simulink model "TosAeb"  (R2021a)
settings: Solver=FixedStepDiscrete  StartTime=0.0  StopTime=inf  FixedStep=0.01  RelTol=1e-3
undefined symbols (not defined in the file or in its folder): ACCModSts, AccSts1, AEB_ObjectMovingOrientation, AEB_ObjectMovingState, CN_BTCActive, CN_CurrentGearR, CN_CurrentGearR_DHT, CN_PTCActive, CN_VDCActive, CN_VSC_EspOff, CN_VSC_FCW_AEB_Error, CN_VSC_FCW_AEB_NoDispay, CN_VSC_FCW_AEB_PerfmDgdtn, CN_VSC_FCW_AEB_TempUnAvlbl, CN_VSC_VehStandstill, CNaccelAvoidMax, CNaccelAvoidMin, CNbrakePedalPress, CNcomfortStrLeftAccelMax, CNcomfortStrLeftAccelMin, CNcomfortStrRightAccelMax, CNcomfortStrRightAccelMin, CNDrvDoorOpen, CNDrvModeEco, CNDrvModeNorm, CNDrvModeOther, CNDrvModeSport, CNDrvSBRreminder, CNNull, CNobjInvalidID, CNPassDoorOpen, CNPassSBRreminder, CNPEBSAccelNegInf, CNPEBSAccelNull, CNPEBSTimeInf, CNPEBSTimeNull, CNSTOPMAX, CNSTOPNULL, CNsubjWidth, CNSwtOFF, CNSwtON, CNTTCMAX, CNTTCNULL, CNVehConfigAEBCycNone, CNVehConfigAEBNone, CNVehConfigAEBPedNone, CNVehConfigFCWNone, CNVehConfigJANone, CollisionSeverity, FALSE, FCWSnvty, JACycObjCalcInfoDefaultParams, JAPedObjCalcInfoDefaultParams, JAVehObjCalcInfoDefaultParams, latCycObjCalcInfoDefaultParams, latPedObjCalcInfoDefaultParams, longObjCalcInfoDefaultParams, ModSts, movingStatus, ObjectType ... (+1112)
libraries: simulink x3031, AEBSFunctionLib x50, Ta_Library x33, AEBSStateMachineLib x23, Mylibrary x12, simulink_extras x7, ObjSelect_lib x3, dspstat3 x2
(part 63 of 102)

== system TosAeb/FcwAebJa_Step_sys/ALG_AEBSystem/ALG_AEBController/AEB_LatObjInfoCalcApp/LatPedObjCalc/LatPedObjCalc/latPedObjInfoCalc/ObjColiisionRiskProbCalc/CollisionRiskProbCalc/EgoReginCorrCalc ==
  dxReginEgoRU : Inport  OutDataTypeStr=single
  dyReginEgoRU : Inport  Port=2
  dxReginEgoLL : Inport  Port=3  OutDataTypeStr=single
  dyReginEgoLL : Inport  Port=4
  Add : Sum  OutDataTypeStr=single
  Add1 : Sum  Inputs=+-  OutDataTypeStr=single
  Add2 : Sum  OutDataTypeStr=single
  ObjectLength : Constant  Value=0.5  OutDataTypeStr=single
  ObjectWidth : Constant  Value=0.5  OutDataTypeStr=single
  dxReginEgoRUCorr : Outport
  dyReginEgoRUCorr : Outport  Port=2
  dyReginEgoLLCorr : Outport  Port=3
  dxReginEgoLLCorr : Outport  Port=4
  wires:
  Add/1 -> dxReginEgoRUCorr/1
  dyReginEgoRU/1 -> Add1/1
  ObjectWidth/1 -> Add2/1, Add1/2
  Add1/1 -> dyReginEgoRUCorr/1
  dxReginEgoLL/1 -> dxReginEgoLLCorr/1
  dyReginEgoLL/1 -> Add2/2
  Add2/1 -> dyReginEgoLLCorr/1
  dxReginEgoRU/1 -> Add/2
  ObjectLength/1 -> Add/1

== system TosAeb/FcwAebJa_Step_sys/ALG_AEBSystem/ALG_AEBController/AEB_LatObjInfoCalcApp/LatPedObjCalc/LatPedObjCalc/latPedObjInfoCalc/ObjColiisionRiskProbCalc/CollisionRiskProbCalc/ObjAbsInfoCalc ==
  vx : Inport
  vy : Inport  Port=2
  dy : Inport  Port=3
  dxFrontBumper : Inport  Port=4
  subjYawRate : Inport  Port=5
  subjVel : Inport  Port=6
  Bus Creator1 : BusCreator  Inputs=2
  RadicalRelevantCalc : Subsystem
  RadicalToXYTrans : Subsystem
  XYToRadicalTrans : Subsystem
  sincosCoefCalc : Subsystem
  velAbsObjCorr : Outport
  wires:
  RadicalRelevantCalc/1(radialVelRel) -> XYToRadicalTrans/2(radialVelRel)  "radialVelRel"
  subjYawRate/1 -> RadicalRelevantCalc/3(subjYawRate)
  dy/1 -> RadicalRelevantCalc/1(dyTargetObjCalc)
  sincosCoefCalc/1(sincosCoef) -> XYToRadicalTrans/3(sincosCoef), RadicalToXYTrans/3(sincosCoef)
  vx/1 -> Bus Creator1/1  "vxRelTargetObjCalc"
  subjVel/1 -> RadicalToXYTrans/1(subjVel)
  dxFrontBumper/1 -> RadicalRelevantCalc/2(dxTargetObjCalc)
  Bus Creator1/1 -> XYToRadicalTrans/1(LongLatVelRel)  "LongLatVelRel"
  vy/1 -> Bus Creator1/2  "vyRelTargetObjCalc"
  XYToRadicalTrans/1(velRadicalXY) -> RadicalToXYTrans/2(velRadicalXY)
  RadicalRelevantCalc/2(objAngleToRear) -> sincosCoefCalc/1(angle)  "objAngleToRear"
  RadicalToXYTrans/1(velAbsObjCorr) -> velAbsObjCorr/1

== system TosAeb/FcwAebJa_Step_sys/ALG_AEBSystem/ALG_AEBController/AEB_LatObjInfoCalcApp/LatPedObjCalc/LatPedObjCalc/latPedObjInfoCalc/ObjColiisionRiskProbCalc/CollisionRiskProbCalc/ObjReginCorrCalc ==
  velAbsObjCorr : Inport
  tPredTime : Inport  Port=2  OutDataTypeStr=single
  ObjectTypeCameraConfirmed : Inport  Port=3
  ObjectLength : Inport  Port=4
  ObjectWidth : Inport  Port=5
  ObjectYawAngle : Inport  Port=6
  ObjectMovingOrientation : Inport  Port=7
  dx : Inport  Port=8
  dy : Inport  Port=9
  AccelXYMaxMinCorrCalc : Subsystem
  ObjFrameCalc : Subsystem
  ObjLengthWidthCalc : Subsystem
  ObjReginCorrCalc : Subsystem
  PedMaxAccelCalc : Subsystem
  dxReginObjRUCorr : Outport
  dyReginObjRUCorr : Outport  Port=2
  dxReginObjLLCorr : Outport  Port=3
  dyReginObjLLCorr : Outport  Port=4
  wires:
  AccelXYMaxMinCorrCalc/1(AccelXMaxMinCorr) -> ObjReginCorrCalc/1(AccelXMaxMinCorr)
  velAbsObjCorr/1 -> PedMaxAccelCalc/1(velAbsObjCorr), AccelXYMaxMinCorrCalc/2(velAbsTargetCorr), ObjReginCorrCalc/3(velAbsTargetCorr)
  PedMaxAccelCalc/1(maxAccelDecel) -> AccelXYMaxMinCorrCalc/1(maxAccelDecel)
  ObjFrameCalc/1(dxMax) -> ObjReginCorrCalc/4(dxMax)
  ObjFrameCalc/3(dyMax) -> ObjReginCorrCalc/6(dyMax)
  AccelXYMaxMinCorrCalc/2(AccelYMaxMinCorr) -> ObjReginCorrCalc/2(AccelYMaxMinCorr)
  tPredTime/1 -> PedMaxAccelCalc/2(tPredTime), [tPredTime]
  ObjFrameCalc/2(dxMin) -> ObjReginCorrCalc/5(dxMin)
  ObjFrameCalc/4(dyMin) -> ObjReginCorrCalc/7(dyMin)
  [tPredTime] -> ObjReginCorrCalc/8(tPredTime)
  dx/1 -> ObjFrameCalc/1(dx)
  dy/1 -> ObjFrameCalc/2(dy)
  ObjLengthWidthCalc/1(ObjLengthCorr) -> ObjFrameCalc/3(ObjLengthCorr)
  ObjLengthWidthCalc/2(ObjWidthCorr) -> ObjFrameCalc/4(ObjWidthCorr)
  ObjReginCorrCalc/1(dxReginObjRUCorr) -> dxReginObjRUCorr/1
  ObjReginCorrCalc/2(dyReginObjRUCorr) -> dyReginObjRUCorr/1
  ObjReginCorrCalc/3(dxReginObjLLCorr) -> dxReginObjLLCorr/1
  ObjReginCorrCalc/4(dyReginObjLLCorr) -> dyReginObjLLCorr/1
  ObjectMovingOrientation/1 -> ObjLengthWidthCalc/5(ObjectMovingOrientation)
  ObjectYawAngle/1 -> ObjLengthWidthCalc/4(ObjectYawAngle)
  ObjectWidth/1 -> ObjLengthWidthCalc/3(ObjectWidth)
  ObjectLength/1 -> ObjLengthWidthCalc/2(ObjectLength)
  ObjectTypeCameraConfirmed/1 -> ObjLengthWidthCalc/1(ObjectTypeCameraConfirmed)

== system TosAeb/FcwAebJa_Step_sys/ALG_AEBSystem/ALG_AEBController/AEB_LatObjInfoCalcApp/LatPedObjCalc/LatPedObjCalc/latPedObjInfoCalc/ObjCrossFlgCal/PedPreCollisionCalcFunc/ObjBoundingBox ==
  ObjLengthCorr : Inport  OutDataTypeStr=single
  ObjWidthCorr : Inport  Port=2  OutDataTypeStr=single
  dxPreRefPointObj : Inport  Port=3  OutDataTypeStr=single
  dyPreRefPointObj : Inport  Port=4  OutDataTypeStr=single
  sincosCoef : Inport  Port=5
  Bus Creator : BusCreator  Inputs=5
  Mux : Mux
  Mux1 : Mux
  ObjLLCoordinate : Subsystem
  ObjLUCoordinate : Subsystem
  ObjRLCoordinate : Subsystem
  ObjRUCoordinate : Subsystem
  objDxPre : Outport
  objDyPre : Outport  Port=2
  wires:
  ObjLengthCorr/1 -> Bus Creator/1  "ObjLengthCorr"
  ObjWidthCorr/1 -> Bus Creator/2  "ObjWidthCorr"
  dxPreRefPointObj/1 -> Bus Creator/3  "dxPreRefPointObj"
  dyPreRefPointObj/1 -> Bus Creator/4  "dyPreRefPointObj"
  sincosCoef/1 -> Bus Creator/5  "sincosCoef"
  Bus Creator/1 -> ObjRLCoordinate/1(inputSignals), ObjRUCoordinate/1(inputSignals), ObjLLCoordinate/1(inputSignals), ObjLUCoordinate/1(inputSignals)
  ObjLUCoordinate/1(dxPreLUObj) -> [dxPreLUObj]  "dxPreLUObj"
  ObjLUCoordinate/2(dyPreLUObj) -> [dyPreLUObj]  "dyPreLUObj"
  ObjLLCoordinate/1(dxPre_LLObj) -> [dxPreLLObj]  "dxPreLLObj"
  ObjLLCoordinate/2(dyPreLLObj) -> [dyPreLLObj]  "dyPreLLObj"
  ObjRUCoordinate/1(dxPreRUObj) -> [dxPreRUObj]  "dxPreRUObj"
  ObjRUCoordinate/2(dyPreRUObj) -> [dyPreRUObj]  "dyPreRUObj"
  ObjRLCoordinate/1(dxPreRLObj) -> [dxPreRLObj]  "dxPreRLObj"
  ObjRLCoordinate/2(dyPreRLObj) -> [dyPreRLObj]  "dyPreRLObj"
  Mux/1 -> objDxPre/1
  Mux1/1 -> objDyPre/1
  [dxPreRUObj] -> Mux/1  "dxPreRUObj"
  [dxPreLUObj] -> Mux/2  "dxPreLUObj"
  [dxPreLLObj] -> Mux/3  "dxPreLLObj"
  [dxPreRLObj] -> Mux/4  "dxPreRLObj"
  [dyPreRUObj] -> Mux1/1  "dyPreRUObj"
  [dyPreLUObj] -> Mux1/2  "dyPreLUObj"
  [dyPreLLObj] -> Mux1/3  "dyPreLLObj"
  [dyPreRLObj] -> Mux1/4  "dyPreRLObj"

== system TosAeb/FcwAebJa_Step_sys/ALG_AEBSystem/ALG_AEBController/AEB_LatObjInfoCalcApp/LatPedObjCalc/LatPedObjCalc/latPedObjInfoCalc/ObjCrossFlgCal/PedPreCollisionCalcFunc/ObjLenthWidthCalc ==
  ObjectYawAngle : Inport
  ObjectMovingOrientation : Inport  Port=2
  ObjectTypeCameraConfirmed : Inport  Port=3
  ObjectLength : Inport  Port=4
  ObjectWidth : Inport  Port=5
  ObjLengthWidthCalc : Stateflow chart  TreatAsAtomicUnit=on
  P_LatPedCA_PreCollisionPedLengthThres : Constant  Value=P_AEB_LatPedCA_PreCollisionPedLengthThres
  P_LatPedCA_PreCollisionPedWidthThres : Constant  Value=P_AEB_LatPedCA_PreCollisionPedWidthThres
  ObjLengthCorr : Outport
  ObjWidthCorr : Outport  Port=2
  wires:
  ObjectYawAngle/1 -> ObjLengthWidthCalc/1(ObjectYawAngle)
  ObjectLength/1 -> ObjLengthWidthCalc/4(ObjectLengthIn)
  ObjectWidth/1 -> ObjLengthWidthCalc/5(ObjectWidthIn)
  ObjLengthWidthCalc/1(ObjectLength) -> ObjLengthCorr/1
  ObjLengthWidthCalc/2(ObjectWidth) -> ObjWidthCorr/1
  ObjectMovingOrientation/1 -> ObjLengthWidthCalc/2(ObjectMovingOrientation)
  P_LatPedCA_PreCollisionPedLengthThres/1 -> ObjLengthWidthCalc/6(P_LatPedCA_PreCollisionPedLengthThres)
  P_LatPedCA_PreCollisionPedWidthThres/1 -> ObjLengthWidthCalc/7(P_LatPedCA_PreCollisionPedWidthThres)
  ObjectTypeCameraConfirmed/1 -> ObjLengthWidthCalc/3(ObjectTypeCameraConfirmed)

== system TosAeb/FcwAebJa_Step_sys/ALG_AEBSystem/ALG_AEBController/AEB_LatObjInfoCalcApp/LatPedObjCalc/LatPedObjCalc/latPedObjInfoCalc/ObjCrossFlgCal/PedPreCollisionCalcFunc/ObjPreReferPointCalc ==
  dx : Inport
  vxAbsObj : Inport  Port=2
  tPredTime : Inport  Port=3  OutDataTypeStr=single
  axAbsObj : Inport  Port=4
  vy : Inport  Port=5
  subjLatAccel : Inport  Port=6
  ay : Inport  Port=7
  dy : Inport  Port=8
  ObjectYawAngle : Inport  Port=9
  Add : Sum  Inputs=+++  OutDataTypeStr=single
  Add1 : Sum  Inputs=+++  OutDataTypeStr=single
  Add2 : Sum
  Degrees to Radians : simulink_extras/Transformations/Degrees to Radians
  Gain : Gain  Gain=0.5  OutDataTypeStr=single
  Gain1 : Gain  Gain=0.5  OutDataTypeStr=single
  Math Function : Math  Operator=square  SignedPower=on  OutDataTypeStr=single  SaturateOnIntegerOverflow=on
  Math Function1 : Math  Operator=square  SignedPower=on  OutDataTypeStr=single  SaturateOnIntegerOverflow=on
  Product{,1..3} (4 alike) : Product  OutDataTypeStr=single
  dxPreRefPointObj : Outport
  dyPreRefPointObj : Outport  Port=2
  YawAnglePreObj : Outport  Port=3
  wires:
  vxAbsObj/1 -> Product/1
  tPredTime/1 -> Product/2, Math Function1/1, Product2/2, Math Function/1
  Product/1 -> Add/2
  Math Function/1 -> Product1/1
  axAbsObj/1 -> Product1/2
  Product1/1 -> Gain/1
  Gain/1 -> Add/3
  Add/1 -> dxPreRefPointObj/1
  dx/1 -> Add/1
  vy/1 -> Product2/1
  Math Function1/1 -> Product3/1
  Product3/1 -> Gain1/1
  Product2/1 -> Add1/1
  Gain1/1 -> Add1/2
  dy/1 -> Add1/3
  Add1/1 -> dyPreRefPointObj/1
  ObjectYawAngle/1 -> Degrees to Radians/1
  subjLatAccel/1 -> Add2/1
  ay/1 -> Add2/2
  Add2/1 -> Product3/2
  Degrees to Radians/1 -> YawAnglePreObj/1

== system TosAeb/FcwAebJa_Step_sys/ALG_AEBSystem/ALG_AEBController/AEB_LatObjInfoCalcApp/LatPedObjCalc/LatPedObjCalc/latPedObjInfoCalc/ObjCrossFlgCal/PedPreCollisionCalcFunc/PreCrossFlgCalcFlow/VectorCrossAlgorithm ==
  x1 : Inport  OutDataTypeStr=single
  y1 : Inport  Port=2  OutDataTypeStr=single
  x2 : Inport  Port=3  OutDataTypeStr=single
  y2 : Inport  Port=4  OutDataTypeStr=single
  m1 : Inport  Port=5  OutDataTypeStr=single
  n1 : Inport  Port=6  OutDataTypeStr=single
  m2 : Inport  Port=7  OutDataTypeStr=single
  n2 : Inport  Port=8  OutDataTypeStr=single
  f : TriggerPort  TriggerType=function-call  VariantControl=Variant
      settings: CopyFcn=Stateflow.SLINSF.SimulinkMan.removeBlockPreDeleteFcn  PreDeleteFcn=Stateflow.SLINSF.SimulinkMan.unsafePredeleteFcn
  Abs : Abs
  Bus Creator : BusCreator  Inputs=8
  Bus Selector : BusSelector  OutputSignals=x2,x1,n2,n1,y2,y1,m2,m1
  Compare To Constant : simulink/Logic and Bit Operations/Compare To Constant  relop=<  const=1e-6  OutDataTypeStr=boolean
  If : If  IfExpression=u1
  If Action Subsystem : Subsystem  TreatAsAtomicUnit=on
  If Action Subsystem1 : Subsystem  TreatAsAtomicUnit=on
  Merge : Merge
  VectorMulit : Subsystem
  pedPreCollisionFlg : Outport
  wires:
  x1/1 -> Bus Creator/1  "x1"
  y1/1 -> Bus Creator/2  "y1"
  x2/1 -> Bus Creator/3  "x2"
  y2/1 -> Bus Creator/4  "y2"
  m1/1 -> Bus Creator/5  "m1"
  n1/1 -> Bus Creator/6  "n1"
  m2/1 -> Bus Creator/7  "m2"
  n2/1 -> Bus Creator/8  "n2"
  Bus Creator/1 -> If Action Subsystem1/2(Coordinations), Bus Selector/1
  VectorMulit/1(vectorMulit) -> If Action Subsystem1/1(vectorMulit), Abs/1
  Abs/1 -> Compare To Constant/1
  Compare To Constant/1 -> If/1
  If/1 -> If Action Subsystem/Action
  If/2 -> If Action Subsystem1/Action
  If Action Subsystem/1(CrossFlg) -> Merge/1
  If Action Subsystem1/1(CrossFlg) -> Merge/2
  Merge/1 -> pedPreCollisionFlg/1
  Bus Selector/2 -> VectorMulit/2(x1)  "<x1>"
  Bus Selector/1 -> VectorMulit/1(x2)  "<x2>"
  Bus Selector/3 -> VectorMulit/3(n2)  "<n2>"
  Bus Selector/4 -> VectorMulit/4(n1)  "<n1>"
  Bus Selector/5 -> VectorMulit/5(y2)  "<y2>"
  Bus Selector/6 -> VectorMulit/6(y1)  "<y1>"
  Bus Selector/7 -> VectorMulit/7(m2)  "<m2>"
  Bus Selector/8 -> VectorMulit/8(m1)  "<m1>"

== system TosAeb/FcwAebJa_Step_sys/ALG_AEBSystem/ALG_AEBController/AEB_LatObjInfoCalcApp/LatPedObjCalc/LatPedObjCalc/latPedObjInfoCalc/ObjCrossFlgCal/PedPreCollisionCalcFunc/PreRefPointEgoCalc ==
  subjVel : Inport
  tPredTime : Inport  Port=2  OutDataTypeStr=single
  subjLongAccel : Inport  Port=3
  subjLatAccel : Inport  Port=4
  subjYawRate : Inport  Port=5
  Add : Sum  OutDataTypeStr=single
  Gain : Gain  Gain=0.5  OutDataTypeStr=single
  Gain1 : Gain  Gain=0.5  OutDataTypeStr=single
  Math Function : Math  Operator=square  SignedPower=on  OutDataTypeStr=single  SaturateOnIntegerOverflow=on
  Product{,1..3} (4 alike) : Product  OutDataTypeStr=single
  " dxPreRefPoint" : Outport
  " dyPreRefPoint" : Outport  Port=2
  YawAnglePre : Outport  Port=3
  wires:
  Gain1/1 -> " dyPreRefPoint"/1
  Gain/1 -> Add/2
  Product3/1 -> YawAnglePre/1
  subjYawRate/1 -> Product3/2
  Product2/1 -> Gain1/1
  subjLatAccel/1 -> Product2/2
  Add/1 -> " dxPreRefPoint"/1
  subjLongAccel/1 -> Product1/2
  Math Function/1 -> Product1/1, Product2/1
  tPredTime/1 -> Math Function/1, Product3/1, Product/2
  subjVel/1 -> Product/1
  Product/1 -> Add/1
  Product1/1 -> Gain/1

== system TosAeb/FcwAebJa_Step_sys/ALG_AEBSystem/ALG_AEBController/AEB_LatObjInfoCalcApp/LatPedObjCalc/LatPedObjCalc/latPedObjInfoCalc/ObjCrossFlgCal/PedPreCollisionCalcFunc/SubjBoundingBox ==
  dxPreRefPoint : Inport  OutDataTypeStr=single
  dyPreRefPoint : Inport  Port=2  OutDataTypeStr=single
  sincosCoef : Inport  Port=3
  Abs : Abs
  Bus Creator : BusCreator  Inputs=6
  Constant : Constant  Value=P_Global_DxvFrontBumper_m
  Constant1 : Constant  Value=P_Global_DxvRearBumper_m
  Constant2 : Constant  Value=P_Global_VehicleWidthMin_m
  Mux : Mux
  Mux1 : Mux
  SubjLLCoordinate : Subsystem
  SubjLUCoordinate : Subsystem
  SubjRLCoordinate : Subsystem
  SubjRUCoordinate : Subsystem
  subjDxPre : Outport
  subjDyPre : Outport  Port=2
  wires:
  Constant2/1 -> Bus Creator/6  "subjWidth"
  Abs/1 -> Bus Creator/5  "dxRearBumperToRearAxis"
  Constant/1 -> Bus Creator/4  "dxFrontBumperToRearAxis"
  sincosCoef/1 -> Bus Creator/3  "sincosCoef"
  dyPreRefPoint/1 -> Bus Creator/2  "dyPreRefPoint"
  dxPreRefPoint/1 -> Bus Creator/1  "dxPreRefPoint"
  Bus Creator/1 -> SubjRLCoordinate/1(inputSignals), SubjRUCoordinate/1(inputSignals), SubjLLCoordinate/1(inputSignals), SubjLUCoordinate/1(inputSignals)
  SubjLUCoordinate/1(dxPreLU) -> [dxPreLU]  "dxPreLU"
  SubjLUCoordinate/2(dyPreLU) -> [dyPreLU]  "dyPreLU"
  SubjLLCoordinate/1(dxPreLL) -> [dxPreLL]  "dxPreLL"
  SubjLLCoordinate/2(dyPreLL) -> [dyPreLL]  "dyPreLL"
  SubjRUCoordinate/1(dxPreRU) -> [dxPreRU]  "dxPreRU"
  SubjRUCoordinate/2(dyPreRU) -> [dyPreRU]  "dyPreRU"
  SubjRLCoordinate/1(dxPreRL) -> [dxPreRL]  "dxPreRL"
  SubjRLCoordinate/2(dyPreRL) -> [dyPreRL]  "dyPreRL"
  Mux/1 -> subjDxPre/1
  Mux1/1 -> subjDyPre/1
  [dxPreRU] -> Mux/1
  [dxPreLU] -> Mux/2
  [dxPreLL] -> Mux/3
  [dxPreRL] -> Mux/4
  [dyPreRU] -> Mux1/1
  [dyPreLU] -> Mux1/2
  [dyPreLL] -> Mux1/3
  [dyPreRL] -> Mux1/4
  Constant1/1 -> Abs/1

== system TosAeb/FcwAebJa_Step_sys/ALG_AEBSystem/ALG_AEBController/AEB_LatObjInfoCalcApp/LatPedObjCalc/LatPedObjCalc/latPedObjInfoCalc/ObjCrossFlgCal/PedPreCollisionCalcFunc/sincosCoefCalc ==
  angle : Inport  OutDataTypeStr=single
  Bus Creator : BusCreator  Inputs=2
  Sincos : Trigonometry  Operator=sincos
  sincosCoef : Outport
  wires:
  Sincos/1 -> Bus Creator/1  "sinCoef"
  angle/1 -> Sincos/1
  Sincos/2 -> Bus Creator/2  "cosCoef"
  Bus Creator/1 -> sincosCoef/1  "sincosCoef"

== system TosAeb/FcwAebJa_Step_sys/ALG_AEBSystem/ALG_AEBController/AEB_LatObjInfoCalcApp/LatPedObjCalc/LatPedObjCalc/latPedObjInfoCalc/ObjCrossFlgCal/PedPreCollisionCalcFunc/sincosCoefCalc1 ==
  angle : Inport
  Bus Creator : BusCreator  Inputs=2
  Sincos : Trigonometry  Operator=sincos
  sincosCoef : Outport
  wires:
  Bus Creator/1 -> sincosCoef/1  "sincosCoef"
  Sincos/2 -> Bus Creator/2  "cosCoef"
  angle/1 -> Sincos/1
  Sincos/1 -> Bus Creator/1  "sinCoef"

== system TosAeb/FcwAebJa_Step_sys/ALG_AEBSystem/ALG_AEBController/AEB_LatObjInfoCalcApp/LatPedObjCalc/Subsystem/latPedObjInfoCalc/ObjColiisionRiskProbCalc/CollisionRiskProbCalc/CollisionRiskProbCalc ==
  dxReginEgoRUCorr : Inport  OutDataTypeStr=single
  dyReginEgoRUCorr : Inport  Port=2  OutDataTypeStr=single
  dyReginEgoLLCorr : Inport  Port=3  OutDataTypeStr=single
  dxReginEgoLLCorr : Inport  Port=4  OutDataTypeStr=single
  dxReginObjRUCorr : Inport  Port=5  OutDataTypeStr=single
  dyReginObjRUCorr : Inport  Port=6  OutDataTypeStr=single
  dxReginObjLLCorr : Inport  Port=7  OutDataTypeStr=single
  dyReginObjLLCorr : Inport  Port=8  OutDataTypeStr=single
  Bus Creator : BusCreator  Inputs=8
  Bus Selector : BusSelector  OutputSignals=dyReginEgoRUCorr,dyReginEgoLLCorr,dyReginObjRUCorr,dyReginObjLLCorr
  Bus Selector1 : BusSelector  OutputSignals=dxReginEgoRUCorr,dxReginEgoLLCorr,dxReginObjRUCorr,dxReginObjLLCorr
  Bus Selector2 : BusSelector  OutputSignals=dxReginObjLLCorr,dyReginObjRUCorr,dyReginObjLLCorr
  CriticalityPointCalc : Subsystem
  dxOverlapFlow : Stateflow chart  TreatAsAtomicUnit=on
  dyOverlapFlow : Stateflow chart  TreatAsAtomicUnit=on
  pedCollisionRiskProbCalc : Subsystem
  dxPreCollison : Outport
  dyPreCollison : Outport  Port=2
  collisionRiskProb : Outport  Port=3
  dyCollisionRiskProb : Outport  Port=4
  dxCollisionRiskProb : Outport  Port=5
  wires:
  dyOverlapFlow/1(dyOverlap) -> pedCollisionRiskProbCalc/1(dyOverlap)
  dyOverlapFlow/2(dyOverlapLimit) -> pedCollisionRiskProbCalc/2(dyOverlapLimit)
  dxOverlapFlow/1(dxOverlap) -> pedCollisionRiskProbCalc/3(dxOverlap)
  dxOverlapFlow/2(dxOverlapLimit) -> pedCollisionRiskProbCalc/4(dxOverlapLimit)
  pedCollisionRiskProbCalc/1(collisionRiskProb) -> collisionRiskProb/1
  dyReginEgoLLCorr/1 -> Bus Creator/3  "dyReginEgoLLCorr"
  dyReginEgoRUCorr/1 -> Bus Creator/2  "dyReginEgoRUCorr"
  dxReginEgoLLCorr/1 -> Bus Creator/4  "dxReginEgoLLCorr"
  dxReginObjRUCorr/1 -> Bus Creator/5  "dxReginObjRUCorr"
  dxReginEgoRUCorr/1 -> Bus Creator/1  "dxReginEgoRUCorr"
  dyReginObjRUCorr/1 -> Bus Creator/6  "dyReginObjRUCorr"
  dxReginObjLLCorr/1 -> Bus Creator/7  "dxReginObjLLCorr"
  dyReginObjLLCorr/1 -> Bus Creator/8  "dyReginObjLLCorr"
  Bus Creator/1 -> Bus Selector1/1, Bus Selector/1, Bus Selector2/1
  Bus Selector/1 -> dyOverlapFlow/1(dyReginEgoRUCorr)  "<dyReginEgoRUCorr>"
  Bus Selector/2 -> dyOverlapFlow/2(dyReginEgoLLCorr)  "<dyReginEgoLLCorr>"
  Bus Selector1/1 -> dxOverlapFlow/1(dxReginEgoRUCorr)  "<dxReginEgoRUCorr>"
  Bus Selector1/2 -> dxOverlapFlow/2(dxReginEgoLLCorr)  "<dxReginEgoLLCorr>"
  Bus Selector2/1 -> CriticalityPointCalc/1(dxReginObjLLCorr)  "<dxReginObjLLCorr>"
  Bus Selector2/2 -> CriticalityPointCalc/2(dyReginObjRUCorr)  "<dyReginObjRUCorr>"
  Bus Selector2/3 -> CriticalityPointCalc/3(dyReginObjLLCorr)  "<dyReginObjLLCorr>"
  CriticalityPointCalc/1(dxPreCollison) -> dxPreCollison/1
  CriticalityPointCalc/2(dyPreCollison) -> dyPreCollison/1
  Bus Selector/3 -> dyOverlapFlow/3(dyReginObjRUCorr)  "<dyReginObjRUCorr>"
  Bus Selector/4 -> dyOverlapFlow/4(dyReginObjLLCorr)  "<dyReginObjLLCorr>"
  Bus Selector1/3 -> dxOverlapFlow/3(dxReginObjRUCorr)  "<dxReginObjRUCorr>"
  Bus Selector1/4 -> dxOverlapFlow/4(dxReginObjLLCorr)  "<dxReginObjLLCorr>"
  pedCollisionRiskProbCalc/2(dyCollisionRiskProb) -> dyCollisionRiskProb/1
  pedCollisionRiskProbCalc/3(dxCollisionRiskProb) -> dxCollisionRiskProb/1

== system TosAeb/FcwAebJa_Step_sys/ALG_AEBSystem/ALG_AEBController/AEB_LatObjInfoCalcApp/LatPedObjCalc/Subsystem/latPedObjInfoCalc/ObjColiisionRiskProbCalc/CollisionRiskProbCalc/EgoReginCalc ==
  tPredTime : Inport  OutDataTypeStr=single
  subjVel : Inport  Port=2
  Abs : Abs
  Add : Sum  OutDataTypeStr=single
  Add1 : Sum  Inputs=+-  OutDataTypeStr=single
  Constant : Constant  Value=P_Global_DxvRearBumper_m
  Constant1 : Constant  Value=P_Global_VehicleWidthMin_m
  Constant2 : Constant  Value=P_Global_DxvFrontBumper_m
  Gain : Gain  Gain=-0.5
  Gain1 : Gain  Gain=0.5
  Product : Product  OutDataTypeStr=single
  dxReginEgoRU : Outport
  dyReginEgoRU : Outport  Port=2
  dxReginEgoLL : Outport  Port=3
  dyReginEgoLL : Outport  Port=4
  wires:
  Product/1 -> Add/1, Add1/1
  Constant2/1 -> Add/2
  Add/1 -> dxReginEgoRU/1
  Constant1/1 -> Gain1/1, Gain/1
  Gain/1 -> dyReginEgoRU/1
  Gain1/1 -> dyReginEgoLL/1
  Constant/1 -> Abs/1
  Add1/1 -> dxReginEgoLL/1
  tPredTime/1 -> Product/1
  subjVel/1 -> Product/2
  Abs/1 -> Add1/2

== system TosAeb/FcwAebJa_Step_sys/ALG_AEBSystem/ALG_AEBController/AEB_LatObjInfoCalcApp/LatPedObjCalc/Subsystem/latPedObjInfoCalc/ObjColiisionRiskProbCalc/CollisionRiskProbCalc/EgoReginCorrCalc ==
  dxReginEgoRU : Inport  OutDataTypeStr=single
  dyReginEgoRU : Inport  Port=2
  dxReginEgoLL : Inport  Port=3  OutDataTypeStr=single
  dyReginEgoLL : Inport  Port=4
  Add : Sum  OutDataTypeStr=single
  Add1 : Sum  Inputs=+-  OutDataTypeStr=single
  Add2 : Sum  OutDataTypeStr=single
  ObjectLength : Constant  Value=0.5  OutDataTypeStr=single
  ObjectWidth : Constant  Value=0.5  OutDataTypeStr=single
  dxReginEgoRUCorr : Outport
  dyReginEgoRUCorr : Outport  Port=2
  dyReginEgoLLCorr : Outport  Port=3
  dxReginEgoLLCorr : Outport  Port=4
  wires:
  Add/1 -> dxReginEgoRUCorr/1
  dyReginEgoRU/1 -> Add1/1
  ObjectWidth/1 -> Add2/1, Add1/2
  Add1/1 -> dyReginEgoRUCorr/1
  dxReginEgoLL/1 -> dxReginEgoLLCorr/1
  dyReginEgoLL/1 -> Add2/2
  Add2/1 -> dyReginEgoLLCorr/1
  dxReginEgoRU/1 -> Add/2
  ObjectLength/1 -> Add/1

== system TosAeb/FcwAebJa_Step_sys/ALG_AEBSystem/ALG_AEBController/AEB_LatObjInfoCalcApp/LatPedObjCalc/Subsystem/latPedObjInfoCalc/ObjColiisionRiskProbCalc/CollisionRiskProbCalc/ObjAbsInfoCalc ==
  vx : Inport
  vy : Inport  Port=2
  dy : Inport  Port=3
  dxFrontBumper : Inport  Port=4
  subjYawRate : Inport  Port=5
  subjVel : Inport  Port=6
  Bus Creator1 : BusCreator  Inputs=2
  RadicalRelevantCalc : Subsystem
  RadicalToXYTrans : Subsystem
  XYToRadicalTrans : Subsystem
  sincosCoefCalc : Subsystem
  velAbsObjCorr : Outport
  wires:
  RadicalRelevantCalc/1(radialVelRel) -> XYToRadicalTrans/2(radialVelRel)  "radialVelRel"
  subjYawRate/1 -> RadicalRelevantCalc/3(subjYawRate)
  dy/1 -> RadicalRelevantCalc/1(dyTargetObjCalc)
  sincosCoefCalc/1(sincosCoef) -> XYToRadicalTrans/3(sincosCoef), RadicalToXYTrans/3(sincosCoef)
  vx/1 -> Bus Creator1/1  "vxRelTargetObjCalc"
  subjVel/1 -> RadicalToXYTrans/1(subjVel)
  dxFrontBumper/1 -> RadicalRelevantCalc/2(dxTargetObjCalc)
  Bus Creator1/1 -> XYToRadicalTrans/1(LongLatVelRel)  "LongLatVelRel"
  vy/1 -> Bus Creator1/2  "vyRelTargetObjCalc"
  XYToRadicalTrans/1(velRadicalXY) -> RadicalToXYTrans/2(velRadicalXY)
  RadicalRelevantCalc/2(objAngleToRear) -> sincosCoefCalc/1(angle)  "objAngleToRear"
  RadicalToXYTrans/1(velAbsObjCorr) -> velAbsObjCorr/1

== system TosAeb/FcwAebJa_Step_sys/ALG_AEBSystem/ALG_AEBController/AEB_LatObjInfoCalcApp/LatPedObjCalc/Subsystem/latPedObjInfoCalc/ObjColiisionRiskProbCalc/CollisionRiskProbCalc/ObjReginCorrCalc ==
  velAbsObjCorr : Inport
  tPredTime : Inport  Port=2  OutDataTypeStr=single
  ObjectTypeCameraConfirmed : Inport  Port=3
  ObjectLength : Inport  Port=4
  ObjectWidth : Inport  Port=5
  ObjectYawAngle : Inport  Port=6
  ObjectMovingOrientation : Inport  Port=7
  dx : Inport  Port=8
  dy : Inport  Port=9
  AccelXYMaxMinCorrCalc : Subsystem
  ObjFrameCalc : Subsystem
  ObjLengthWidthCalc : Subsystem
  ObjReginCorrCalc : Subsystem
  PedMaxAccelCalc : Subsystem
  dxReginObjRUCorr : Outport
  dyReginObjRUCorr : Outport  Port=2
  dxReginObjLLCorr : Outport  Port=3
  dyReginObjLLCorr : Outport  Port=4
  wires:
  AccelXYMaxMinCorrCalc/1(AccelXMaxMinCorr) -> ObjReginCorrCalc/1(AccelXMaxMinCorr)
  velAbsObjCorr/1 -> PedMaxAccelCalc/1(velAbsObjCorr), AccelXYMaxMinCorrCalc/2(velAbsTargetCorr), ObjReginCorrCalc/3(velAbsTargetCorr)
  PedMaxAccelCalc/1(maxAccelDecel) -> AccelXYMaxMinCorrCalc/1(maxAccelDecel)
  ObjFrameCalc/1(dxMax) -> ObjReginCorrCalc/4(dxMax)
  ObjFrameCalc/3(dyMax) -> ObjReginCorrCalc/6(dyMax)
  AccelXYMaxMinCorrCalc/2(AccelYMaxMinCorr) -> ObjReginCorrCalc/2(AccelYMaxMinCorr)
  tPredTime/1 -> PedMaxAccelCalc/2(tPredTime), [tPredTime]
  ObjFrameCalc/2(dxMin) -> ObjReginCorrCalc/5(dxMin)
  ObjFrameCalc/4(dyMin) -> ObjReginCorrCalc/7(dyMin)
  [tPredTime] -> ObjReginCorrCalc/8(tPredTime)
  dx/1 -> ObjFrameCalc/1(dx)
  dy/1 -> ObjFrameCalc/2(dy)
  ObjLengthWidthCalc/1(ObjLengthCorr) -> ObjFrameCalc/3(ObjLengthCorr)
  ObjLengthWidthCalc/2(ObjWidthCorr) -> ObjFrameCalc/4(ObjWidthCorr)
  ObjReginCorrCalc/1(dxReginObjRUCorr) -> dxReginObjRUCorr/1
  ObjReginCorrCalc/2(dyReginObjRUCorr) -> dyReginObjRUCorr/1
  ObjReginCorrCalc/3(dxReginObjLLCorr) -> dxReginObjLLCorr/1
  ObjReginCorrCalc/4(dyReginObjLLCorr) -> dyReginObjLLCorr/1
  ObjectMovingOrientation/1 -> ObjLengthWidthCalc/5(ObjectMovingOrientation)
  ObjectYawAngle/1 -> ObjLengthWidthCalc/4(ObjectYawAngle)
  ObjectWidth/1 -> ObjLengthWidthCalc/3(ObjectWidth)
  ObjectLength/1 -> ObjLengthWidthCalc/2(ObjectLength)
  ObjectTypeCameraConfirmed/1 -> ObjLengthWidthCalc/1(ObjectTypeCameraConfirmed)

== system TosAeb/FcwAebJa_Step_sys/ALG_AEBSystem/ALG_AEBController/AEB_LatObjInfoCalcApp/LatPedObjCalc/Subsystem/latPedObjInfoCalc/ObjCrossFlgCal/PedPreCollisionCalcFunc/ObjBoundingBox ==
  ObjLengthCorr : Inport  OutDataTypeStr=single
  ObjWidthCorr : Inport  Port=2  OutDataTypeStr=single
  dxPreRefPointObj : Inport  Port=3  OutDataTypeStr=single
  dyPreRefPointObj : Inport  Port=4  OutDataTypeStr=single
  sincosCoef : Inport  Port=5
  Bus Creator : BusCreator  Inputs=5
  Mux : Mux
  Mux1 : Mux
  ObjLLCoordinate : Subsystem
  ObjLUCoordinate : Subsystem
  ObjRLCoordinate : Subsystem
  ObjRUCoordinate : Subsystem
  objDxPre : Outport
  objDyPre : Outport  Port=2
  wires:
  ObjLengthCorr/1 -> Bus Creator/1  "ObjLengthCorr"
  ObjWidthCorr/1 -> Bus Creator/2  "ObjWidthCorr"
  dxPreRefPointObj/1 -> Bus Creator/3  "dxPreRefPointObj"
  dyPreRefPointObj/1 -> Bus Creator/4  "dyPreRefPointObj"
  sincosCoef/1 -> Bus Creator/5  "sincosCoef"
  Bus Creator/1 -> ObjRLCoordinate/1(inputSignals), ObjRUCoordinate/1(inputSignals), ObjLLCoordinate/1(inputSignals), ObjLUCoordinate/1(inputSignals)
  ObjLUCoordinate/1(dxPreLUObj) -> [dxPreLUObj]  "dxPreLUObj"
  ObjLUCoordinate/2(dyPreLUObj) -> [dyPreLUObj]  "dyPreLUObj"
  ObjLLCoordinate/1(dxPre_LLObj) -> [dxPreLLObj]  "dxPreLLObj"
  ObjLLCoordinate/2(dyPreLLObj) -> [dyPreLLObj]  "dyPreLLObj"
  ObjRUCoordinate/1(dxPreRUObj) -> [dxPreRUObj]  "dxPreRUObj"
  ObjRUCoordinate/2(dyPreRUObj) -> [dyPreRUObj]  "dyPreRUObj"
  ObjRLCoordinate/1(dxPreRLObj) -> [dxPreRLObj]  "dxPreRLObj"
  ObjRLCoordinate/2(dyPreRLObj) -> [dyPreRLObj]  "dyPreRLObj"
  Mux/1 -> objDxPre/1
  Mux1/1 -> objDyPre/1
  [dxPreRUObj] -> Mux/1  "dxPreRUObj"
  [dxPreLUObj] -> Mux/2  "dxPreLUObj"
  [dxPreLLObj] -> Mux/3  "dxPreLLObj"
  [dxPreRLObj] -> Mux/4  "dxPreRLObj"
  [dyPreRUObj] -> Mux1/1  "dyPreRUObj"
  [dyPreLUObj] -> Mux1/2  "dyPreLUObj"
  [dyPreLLObj] -> Mux1/3  "dyPreLLObj"
  [dyPreRLObj] -> Mux1/4  "dyPreRLObj"

== system TosAeb/FcwAebJa_Step_sys/ALG_AEBSystem/ALG_AEBController/AEB_LatObjInfoCalcApp/LatPedObjCalc/Subsystem/latPedObjInfoCalc/ObjCrossFlgCal/PedPreCollisionCalcFunc/ObjLenthWidthCalc ==
  ObjectYawAngle : Inport
  ObjectMovingOrientation : Inport  Port=2
  ObjectTypeCameraConfirmed : Inport  Port=3
  ObjectLength : Inport  Port=4
  ObjectWidth : Inport  Port=5
  ObjLengthWidthCalc : Stateflow chart  TreatAsAtomicUnit=on
  P_LatPedCA_PreCollisionPedLengthThres : Constant  Value=P_AEB_LatPedCA_PreCollisionPedLengthThres
  P_LatPedCA_PreCollisionPedWidthThres : Constant  Value=P_AEB_LatPedCA_PreCollisionPedWidthThres
  ObjLengthCorr : Outport
  ObjWidthCorr : Outport  Port=2
  wires:
  ObjectYawAngle/1 -> ObjLengthWidthCalc/1(ObjectYawAngle)
  ObjectLength/1 -> ObjLengthWidthCalc/4(ObjectLengthIn)
  ObjectWidth/1 -> ObjLengthWidthCalc/5(ObjectWidthIn)
  ObjLengthWidthCalc/1(ObjectLength) -> ObjLengthCorr/1
  ObjLengthWidthCalc/2(ObjectWidth) -> ObjWidthCorr/1
  ObjectMovingOrientation/1 -> ObjLengthWidthCalc/2(ObjectMovingOrientation)
  P_LatPedCA_PreCollisionPedLengthThres/1 -> ObjLengthWidthCalc/6(P_LatPedCA_PreCollisionPedLengthThres)
  P_LatPedCA_PreCollisionPedWidthThres/1 -> ObjLengthWidthCalc/7(P_LatPedCA_PreCollisionPedWidthThres)
  ObjectTypeCameraConfirmed/1 -> ObjLengthWidthCalc/3(ObjectTypeCameraConfirmed)

== system TosAeb/FcwAebJa_Step_sys/ALG_AEBSystem/ALG_AEBController/AEB_LatObjInfoCalcApp/LatPedObjCalc/Subsystem/latPedObjInfoCalc/ObjCrossFlgCal/PedPreCollisionCalcFunc/ObjPreReferPointCalc ==
  dx : Inport
  vxAbsObj : Inport  Port=2
  tPredTime : Inport  Port=3  OutDataTypeStr=single
  axAbsObj : Inport  Port=4
  vy : Inport  Port=5
  subjLatAccel : Inport  Port=6
  ay : Inport  Port=7
  dy : Inport  Port=8
  ObjectYawAngle : Inport  Port=9
  Add : Sum  Inputs=+++  OutDataTypeStr=single
  Add1 : Sum  Inputs=+++  OutDataTypeStr=single
  Add2 : Sum
  Degrees to Radians : simulink_extras/Transformations/Degrees to Radians
  Gain : Gain  Gain=0.5  OutDataTypeStr=single
  Gain1 : Gain  Gain=0.5  OutDataTypeStr=single
  Math Function : Math  Operator=square  SignedPower=on  OutDataTypeStr=single  SaturateOnIntegerOverflow=on
  Math Function1 : Math  Operator=square  SignedPower=on  OutDataTypeStr=single  SaturateOnIntegerOverflow=on
  Product{,1..3} (4 alike) : Product  OutDataTypeStr=single
  dxPreRefPointObj : Outport
  dyPreRefPointObj : Outport  Port=2
  YawAnglePreObj : Outport  Port=3
  wires:
  vxAbsObj/1 -> Product/1
  tPredTime/1 -> Product/2, Math Function1/1, Product2/2, Math Function/1
  Product/1 -> Add/2
  Math Function/1 -> Product1/1
  axAbsObj/1 -> Product1/2
  Product1/1 -> Gain/1
  Gain/1 -> Add/3
  Add/1 -> dxPreRefPointObj/1
  dx/1 -> Add/1
  vy/1 -> Product2/1
  Math Function1/1 -> Product3/1
  Product3/1 -> Gain1/1
  Product2/1 -> Add1/1
  Gain1/1 -> Add1/2
  dy/1 -> Add1/3
  Add1/1 -> dyPreRefPointObj/1
  ObjectYawAngle/1 -> Degrees to Radians/1
  subjLatAccel/1 -> Add2/1
  ay/1 -> Add2/2
  Add2/1 -> Product3/2
  Degrees to Radians/1 -> YawAnglePreObj/1

== system TosAeb/FcwAebJa_Step_sys/ALG_AEBSystem/ALG_AEBController/AEB_LatObjInfoCalcApp/LatPedObjCalc/Subsystem/latPedObjInfoCalc/ObjCrossFlgCal/PedPreCollisionCalcFunc/PreCrossFlgCalcFlow/VectorCrossAlgorithm ==
  x1 : Inport  OutDataTypeStr=single
  y1 : Inport  Port=2  OutDataTypeStr=single
  x2 : Inport  Port=3  OutDataTypeStr=single
  y2 : Inport  Port=4  OutDataTypeStr=single
  m1 : Inport  Port=5  OutDataTypeStr=single
  n1 : Inport  Port=6  OutDataTypeStr=single
  m2 : Inport  Port=7  OutDataTypeStr=single
  n2 : Inport  Port=8  OutDataTypeStr=single
  f : TriggerPort  TriggerType=function-call  VariantControl=Variant
      settings: CopyFcn=Stateflow.SLINSF.SimulinkMan.removeBlockPreDeleteFcn  PreDeleteFcn=Stateflow.SLINSF.SimulinkMan.unsafePredeleteFcn
  Abs : Abs
  Bus Creator : BusCreator  Inputs=8
  Bus Selector : BusSelector  OutputSignals=x2,x1,n2,n1,y2,y1,m2,m1
  Compare To Constant : simulink/Logic and Bit Operations/Compare To Constant  relop=<  const=1e-6  OutDataTypeStr=boolean
  If : If  IfExpression=u1
  If Action Subsystem : Subsystem  TreatAsAtomicUnit=on
  If Action Subsystem1 : Subsystem  TreatAsAtomicUnit=on
  Merge : Merge
  VectorMulit : Subsystem
  pedPreCollisionFlg : Outport
  wires:
  x1/1 -> Bus Creator/1  "x1"
  y1/1 -> Bus Creator/2  "y1"
  x2/1 -> Bus Creator/3  "x2"
  y2/1 -> Bus Creator/4  "y2"
  m1/1 -> Bus Creator/5  "m1"
  n1/1 -> Bus Creator/6  "n1"
  m2/1 -> Bus Creator/7  "m2"
  n2/1 -> Bus Creator/8  "n2"
  Bus Creator/1 -> If Action Subsystem1/2(Coordinations), Bus Selector/1
  VectorMulit/1(vectorMulit) -> If Action Subsystem1/1(vectorMulit), Abs/1
  Abs/1 -> Compare To Constant/1
  Compare To Constant/1 -> If/1
  If/1 -> If Action Subsystem/Action
  If/2 -> If Action Subsystem1/Action
  If Action Subsystem/1(CrossFlg) -> Merge/1
  If Action Subsystem1/1(CrossFlg) -> Merge/2
  Merge/1 -> pedPreCollisionFlg/1
  Bus Selector/2 -> VectorMulit/2(x1)  "<x1>"
  Bus Selector/1 -> VectorMulit/1(x2)  "<x2>"
  Bus Selector/3 -> VectorMulit/3(n2)  "<n2>"
  Bus Selector/4 -> VectorMulit/4(n1)  "<n1>"
  Bus Selector/5 -> VectorMulit/5(y2)  "<y2>"
  Bus Selector/6 -> VectorMulit/6(y1)  "<y1>"
  Bus Selector/7 -> VectorMulit/7(m2)  "<m2>"
  Bus Selector/8 -> VectorMulit/8(m1)  "<m1>"

== system TosAeb/FcwAebJa_Step_sys/ALG_AEBSystem/ALG_AEBController/AEB_LatObjInfoCalcApp/LatPedObjCalc/Subsystem/latPedObjInfoCalc/ObjCrossFlgCal/PedPreCollisionCalcFunc/PreRefPointEgoCalc ==
  subjVel : Inport
  tPredTime : Inport  Port=2  OutDataTypeStr=single
  subjLongAccel : Inport  Port=3
  subjLatAccel : Inport  Port=4
  subjYawRate : Inport  Port=5
  Add : Sum  OutDataTypeStr=single
  Gain : Gain  Gain=0.5  OutDataTypeStr=single
  Gain1 : Gain  Gain=0.5  OutDataTypeStr=single
  Math Function : Math  Operator=square  SignedPower=on  OutDataTypeStr=single  SaturateOnIntegerOverflow=on
  Product{,1..3} (4 alike) : Product  OutDataTypeStr=single
  " dxPreRefPoint" : Outport
  " dyPreRefPoint" : Outport  Port=2
  YawAnglePre : Outport  Port=3
  wires:
  Gain1/1 -> " dyPreRefPoint"/1
  Gain/1 -> Add/2
  Product3/1 -> YawAnglePre/1
  subjYawRate/1 -> Product3/2
  Product2/1 -> Gain1/1
  subjLatAccel/1 -> Product2/2
  Add/1 -> " dxPreRefPoint"/1
  subjLongAccel/1 -> Product1/2
  Math Function/1 -> Product1/1, Product2/1
  tPredTime/1 -> Math Function/1, Product3/1, Product/2
  subjVel/1 -> Product/1
  Product/1 -> Add/1
  Product1/1 -> Gain/1

== system TosAeb/FcwAebJa_Step_sys/ALG_AEBSystem/ALG_AEBController/AEB_LatObjInfoCalcApp/LatPedObjCalc/Subsystem/latPedObjInfoCalc/ObjCrossFlgCal/PedPreCollisionCalcFunc/SubjBoundingBox ==
  dxPreRefPoint : Inport  OutDataTypeStr=single
  dyPreRefPoint : Inport  Port=2  OutDataTypeStr=single
  sincosCoef : Inport  Port=3
  Abs : Abs
  Bus Creator : BusCreator  Inputs=6
  Constant : Constant  Value=P_Global_DxvFrontBumper_m
  Constant1 : Constant  Value=P_Global_DxvRearBumper_m
  Constant2 : Constant  Value=P_Global_VehicleWidthMin_m
  Mux : Mux
  Mux1 : Mux
  SubjLLCoordinate : Subsystem
  SubjLUCoordinate : Subsystem
  SubjRLCoordinate : Subsystem
  SubjRUCoordinate : Subsystem
  subjDxPre : Outport
  subjDyPre : Outport  Port=2
  wires:
  Constant2/1 -> Bus Creator/6  "subjWidth"
  Abs/1 -> Bus Creator/5  "dxRearBumperToRearAxis"
  Constant/1 -> Bus Creator/4  "dxFrontBumperToRearAxis"
  sincosCoef/1 -> Bus Creator/3  "sincosCoef"
  dyPreRefPoint/1 -> Bus Creator/2  "dyPreRefPoint"
  dxPreRefPoint/1 -> Bus Creator/1  "dxPreRefPoint"
  Bus Creator/1 -> SubjRLCoordinate/1(inputSignals), SubjRUCoordinate/1(inputSignals), SubjLLCoordinate/1(inputSignals), SubjLUCoordinate/1(inputSignals)
  SubjLUCoordinate/1(dxPreLU) -> [dxPreLU]  "dxPreLU"
  SubjLUCoordinate/2(dyPreLU) -> [dyPreLU]  "dyPreLU"
  SubjLLCoordinate/1(dxPreLL) -> [dxPreLL]  "dxPreLL"
  SubjLLCoordinate/2(dyPreLL) -> [dyPreLL]  "dyPreLL"
  SubjRUCoordinate/1(dxPreRU) -> [dxPreRU]  "dxPreRU"
  SubjRUCoordinate/2(dyPreRU) -> [dyPreRU]  "dyPreRU"
  SubjRLCoordinate/1(dxPreRL) -> [dxPreRL]  "dxPreRL"
  SubjRLCoordinate/2(dyPreRL) -> [dyPreRL]  "dyPreRL"
  Mux/1 -> subjDxPre/1
  Mux1/1 -> subjDyPre/1
  [dxPreRU] -> Mux/1
  [dxPreLU] -> Mux/2
  [dxPreLL] -> Mux/3
  [dxPreRL] -> Mux/4
  [dyPreRU] -> Mux1/1
  [dyPreLU] -> Mux1/2
  [dyPreLL] -> Mux1/3
  [dyPreRL] -> Mux1/4
  Constant1/1 -> Abs/1

== system TosAeb/FcwAebJa_Step_sys/ALG_AEBSystem/ALG_AEBController/AEB_LatObjInfoCalcApp/LatPedObjCalc/Subsystem/latPedObjInfoCalc/ObjCrossFlgCal/PedPreCollisionCalcFunc/sincosCoefCalc ==
  angle : Inport  OutDataTypeStr=single
  Bus Creator : BusCreator  Inputs=2
  Sincos : Trigonometry  Operator=sincos
  sincosCoef : Outport
  wires:
  Sincos/1 -> Bus Creator/1  "sinCoef"
  angle/1 -> Sincos/1
  Sincos/2 -> Bus Creator/2  "cosCoef"
  Bus Creator/1 -> sincosCoef/1  "sincosCoef"

== system TosAeb/FcwAebJa_Step_sys/ALG_AEBSystem/ALG_AEBController/AEB_LatObjInfoCalcApp/LatPedObjCalc/Subsystem/latPedObjInfoCalc/ObjCrossFlgCal/PedPreCollisionCalcFunc/sincosCoefCalc1 ==
  angle : Inport
  Bus Creator : BusCreator  Inputs=2
  Sincos : Trigonometry  Operator=sincos
  sincosCoef : Outport
  wires:
  Bus Creator/1 -> sincosCoef/1  "sincosCoef"
  Sincos/2 -> Bus Creator/2  "cosCoef"
  angle/1 -> Sincos/1
  Sincos/1 -> Bus Creator/1  "sinCoef"

== system TosAeb/FcwAebJa_Step_sys/ALG_AEBSystem/ALG_AEBController/AEB_LongTgtInfoCalc/LongObjCalcInfoCalc/AEBS_LongCollisionAssesApp/LatCollisionAvoidCalc/AccelLatAvoidCalc4SO/accelLatAvoidLeftCalc/CalculateAccelSteeringLeft ==
  note: if  PcomfortStrLatDistMinSO^2 < (dxRelTargetObjCalc^2 + dyRelTargetObjCalc^2)
      | 	tempFlgCalc = 1;
      | else
      | 	tempFlgCalc = 0;
  dxRelTargetObjCalc : Inport
  dyRelTargetObjCalc : Inport  Port=2
  subjVel : Inport  Port=3
  Action Port : ActionPort  ActionPortLabel=else
  Bus Creator : BusCreator  Inputs=3
  Bus Selector : BusSelector  OutputSignals=dxRelTargetObjCalc,dyRelTargetObjCalc
  Bus Selector1 : BusSelector  OutputSignals=dyRelTargetObjCalc
  Bus Selector2 : BusSelector  OutputSignals=dxRelTargetObjCalc,dyRelTargetObjCalc,subjVel
  "CalculateAccelSteeringLeft " : Subsystem  TreatAsAtomicUnit=on
  CalculateAccelSteeringLeftFlow : Stateflow chart  TreatAsAtomicUnit=on
  P_LongCA_LatAvoidLatDistMinSO : Constant  Value=P_AEB_LongCA_LatAvoidLatDistMinSO
  tempFlgCalc : Subsystem
  accelLatAvoidLeft : Outport
  wires:
  Bus Selector2/3 -> "CalculateAccelSteeringLeft "/3(subjVel)  "<subjVel>"
  Bus Selector2/2 -> "CalculateAccelSteeringLeft "/2(dyRelTargetObjCalc)  "<dyRelTargetObjCalc>"
  Bus Selector2/1 -> "CalculateAccelSteeringLeft "/1(dxRelTargetObjCalc)  "<dxRelTargetObjCalc>"
  Bus Selector1/1 -> CalculateAccelSteeringLeftFlow/3(dyRelTargetObjCalc)  "<dyRelTargetObjCalc>"
  Bus Selector/1 -> tempFlgCalc/1(dxRelTargetObjCalc)  "<dxRelTargetObjCalc>"
  Bus Selector/2 -> tempFlgCalc/2(dyRelTargetObjCalc)  "<dyRelTargetObjCalc>"
  Bus Creator/1 -> Bus Selector/1, Bus Selector2/1, Bus Selector1/1
  subjVel/1 -> Bus Creator/3  "subjVel"
  dyRelTargetObjCalc/1 -> Bus Creator/2  "dyRelTargetObjCalc"
  dxRelTargetObjCalc/1 -> Bus Creator/1  "dxRelTargetObjCalc"
  tempFlgCalc/1(tempFlg) -> CalculateAccelSteeringLeftFlow/1(tempFlg)
  P_LongCA_LatAvoidLatDistMinSO/1 -> CalculateAccelSteeringLeftFlow/2(PcomfortStrLatDistMinSO)
  CalculateAccelSteeringLeftFlow/1(accelSteeringLeft) -> accelLatAvoidLeft/1
  "CalculateAccelSteeringLeft "/1(accelLatAvoidLeft) -> CalculateAccelSteeringLeftFlow/4(accelSteeringLeftIn)
  CalculateAccelSteeringLeftFlow/2(accelSteeringLeftCalcFunc()) -> "CalculateAccelSteeringLeft "/Trigger

== system TosAeb/FcwAebJa_Step_sys/ALG_AEBSystem/ALG_AEBController/AEB_LongTgtInfoCalc/LongObjCalcInfoCalc/AEBS_LongCollisionAssesApp/LatCollisionAvoidCalc/AccelLatAvoidCalc4SO/accelLatAvoidLeftCalc/dxMinLeftCalc ==
  note: Calculate the following Formula
  tempData1 : Inport
  tempData2 : Inport  Port=2
  Action Port : ActionPort  ActionPortLabel=else
  Product : Product
  Sqrt : Sqrt  SaturateOnIntegerOverflow=on
  dxMinLeft : Outport
  wires:
  tempData2/1 -> Product/2
  tempData1/1 -> Product/1
  Product/1 -> Sqrt/1
  Sqrt/1 -> dxMinLeft/1

== system TosAeb/FcwAebJa_Step_sys/ALG_AEBSystem/ALG_AEBController/AEB_LongTgtInfoCalc/LongObjCalcInfoCalc/AEBS_LongCollisionAssesApp/LatCollisionAvoidCalc/AccelLatAvoidCalc4SO/accelLatAvoidLeftCalc/dxMinLeftSet0 ==
  Action Port : ActionPort  ActionPortLabel=if(u1 | u2)
  Constant : Constant  Value=CNNull
  dxMinLeft : Outport
  wires:
  Constant/1 -> dxMinLeft/1

== system TosAeb/FcwAebJa_Step_sys/ALG_AEBSystem/ALG_AEBController/AEB_LongTgtInfoCalc/LongObjCalcInfoCalc/AEBS_LongCollisionAssesApp/LatCollisionAvoidCalc/AccelLatAvoidCalc4SO/accelLatAvoidLeftCalc/latAvoidAccelMax ==
  Action Port : ActionPort  ActionPortLabel=if(u1 > u2)
  latAvoidLeftAccelMax : Constant  Value=CNcomfortStrLeftAccelMax
  accelLatAvoidLeft : Outport
  wires:
  latAvoidLeftAccelMax/1 -> accelLatAvoidLeft/1

== system TosAeb/FcwAebJa_Step_sys/ALG_AEBSystem/ALG_AEBController/AEB_LongTgtInfoCalc/LongObjCalcInfoCalc/AEBS_LongCollisionAssesApp/LatCollisionAvoidCalc/AccelLatAvoidCalc4SO/accelLatAvoidLeftCalc/tempDataCalc ==
  dyRelTargetObjCalc : Inport
  Add : Sum
  Compare To Zero : simulink/Logic and Bit Operations/Compare To Zero  relop=<=  OutDataTypeStr=boolean
  Compare To Zero1 : simulink/Logic and Bit Operations/Compare To Zero  relop=<=  OutDataTypeStr=boolean
  Gain : Gain  Gain=2
  P_LongCA_LatAvoidLatDistMinSO : Constant  Value=P_AEB_LongCA_LatAvoidLatDistMinSO
  P_LongCA_LatAvoidStrRminSO : Constant  Value=P_AEB_LongCA_LatAvoidStrRminSO
  Subtract : Sum  Inputs=+-
  Flg1 : Outport
  Flg2 : Outport  Port=2
  tempData1 : Outport  Port=3
  tempData2 : Outport  Port=4
  wires:
  dyRelTargetObjCalc/1 -> Add/2
  P_LongCA_LatAvoidLatDistMinSO/1 -> Add/1
  Add/1 -> Subtract/2, Compare To Zero1/1, tempData2/1
  Subtract/1 -> Compare To Zero/1, tempData1/1
  Compare To Zero/1 -> Flg1/1
  P_LongCA_LatAvoidStrRminSO/1 -> Gain/1
  Compare To Zero1/1 -> Flg2/1
  Gain/1 -> Subtract/1

== system TosAeb/FcwAebJa_Step_sys/ALG_AEBSystem/ALG_AEBController/AEB_LongTgtInfoCalc/LongObjCalcInfoCalc/AEBS_LongCollisionAssesApp/LatCollisionAvoidCalc/AccelLatAvoidCalc4SO/accelLatAvoidRightCalc/CalculateAccelSteeringRight ==
  note: if  PcomfortStrLatDistMinSO^2 < (dxRelTargetObjCalc^2 + dyRelTargetObjCalc^2)
      | 	tempFlgCalc = 1;
      | else
      | 	tempFlgCalc = 0;
  dxRelTargetObjCalc : Inport
  dyRelTargetObjCalc : Inport  Port=2
  subjVel : Inport  Port=3
  Action Port : ActionPort  ActionPortLabel=else
  Bus Creator : BusCreator  Inputs=3
  Bus Selector : BusSelector  OutputSignals=dxRelTargetObjCalc,dyRelTargetObjCalc
  Bus Selector1 : BusSelector  OutputSignals=dyRelTargetObjCalc
  Bus Selector2 : BusSelector  OutputSignals=dxRelTargetObjCalc,dyRelTargetObjCalc,subjVel
  "CalculateAccelSteeringLeft " : Subsystem  TreatAsAtomicUnit=on
  CalculateAccelSteeringLeftFlow : Stateflow chart  TreatAsAtomicUnit=on
  dyMinStationary1 : Constant  Value=PcomfortStrLatDistMinSO
  tempFlgCalc : Subsystem
  accelLatAvoidRight : Outport
  wires:
  CalculateAccelSteeringLeftFlow/1(accelSteeringRight) -> accelLatAvoidRight/1
  Bus Selector2/3 -> "CalculateAccelSteeringLeft "/3(subjVel)  "<subjVel>"
  Bus Selector2/2 -> "CalculateAccelSteeringLeft "/2(dyRelTargetObjCalc)  "<dyRelTargetObjCalc>"
  Bus Selector2/1 -> "CalculateAccelSteeringLeft "/1(dxRelTargetObjCalc)  "<dxRelTargetObjCalc>"
  Bus Selector1/1 -> CalculateAccelSteeringLeftFlow/3(dyRelTargetObjCalc)  "<dyRelTargetObjCalc>"
  Bus Selector/1 -> tempFlgCalc/1(dxRelTargetObjCalc)  "<dxRelTargetObjCalc>"
  Bus Selector/2 -> tempFlgCalc/2(dyRelTargetObjCalc)  "<dyRelTargetObjCalc>"
  Bus Creator/1 -> Bus Selector/1, Bus Selector1/1, Bus Selector2/1
  subjVel/1 -> Bus Creator/3  "subjVel"
  dyRelTargetObjCalc/1 -> Bus Creator/2  "dyRelTargetObjCalc"
  dxRelTargetObjCalc/1 -> Bus Creator/1  "dxRelTargetObjCalc"
  tempFlgCalc/1(tempFlg) -> CalculateAccelSteeringLeftFlow/1(tempFlg)
  dyMinStationary1/1 -> CalculateAccelSteeringLeftFlow/2(PcomfortStrLatDistMinSO)
  "CalculateAccelSteeringLeft "/1(accelLatAvoidRight) -> CalculateAccelSteeringLeftFlow/4(accelSteeringRightIn)
  CalculateAccelSteeringLeftFlow/2(accelSteeringRightCalcFunc()) -> "CalculateAccelSteeringLeft "/Trigger

== system TosAeb/FcwAebJa_Step_sys/ALG_AEBSystem/ALG_AEBController/AEB_LongTgtInfoCalc/LongObjCalcInfoCalc/AEBS_LongCollisionAssesApp/LatCollisionAvoidCalc/AccelLatAvoidCalc4SO/accelLatAvoidRightCalc/dxMinRightCalc ==
  note: Calculate the following Formula
  tempData1 : Inport
  tempData2 : Inport  Port=2
  Action Port : ActionPort  ActionPortLabel=else
  Product : Product
  Sqrt : Sqrt  SaturateOnIntegerOverflow=on
  dxMinRight : Outport
  wires:
  tempData2/1 -> Product/2
  tempData1/1 -> Product/1
  Product/1 -> Sqrt/1
  Sqrt/1 -> dxMinRight/1

== system TosAeb/FcwAebJa_Step_sys/ALG_AEBSystem/ALG_AEBController/AEB_LongTgtInfoCalc/LongObjCalcInfoCalc/AEBS_LongCollisionAssesApp/LatCollisionAvoidCalc/AccelLatAvoidCalc4SO/accelLatAvoidRightCalc/dxMinRightSet0 ==
  Action Port : ActionPort  ActionPortLabel=if(u1 | u2)
  Constant : Constant  Value=CNNull
  dxMinRight : Outport
  wires:
  Constant/1 -> dxMinRight/1

== system TosAeb/FcwAebJa_Step_sys/ALG_AEBSystem/ALG_AEBController/AEB_LongTgtInfoCalc/LongObjCalcInfoCalc/AEBS_LongCollisionAssesApp/LatCollisionAvoidCalc/AccelLatAvoidCalc4SO/accelLatAvoidRightCalc/latAvoidAccelMin ==
  Action Port : ActionPort  ActionPortLabel=if(u1 > u2)
  latAvoidRightAccelMin : Constant  Value=CNcomfortStrRightAccelMin
  accelLatAvoidRight : Outport
  wires:
  latAvoidRightAccelMin/1 -> accelLatAvoidRight/1

== system TosAeb/FcwAebJa_Step_sys/ALG_AEBSystem/ALG_AEBController/AEB_LongTgtInfoCalc/LongObjCalcInfoCalc/AEBS_LongCollisionAssesApp/LatCollisionAvoidCalc/AccelLatAvoidCalc4SO/accelLatAvoidRightCalc/tempDataCalc ==
  dyRelTargetObjCalc : Inport
  Add : Sum  Inputs=+-
  Compare To Zero : simulink/Logic and Bit Operations/Compare To Zero  relop=<=  OutDataTypeStr=boolean
  Compare To Zero1 : simulink/Logic and Bit Operations/Compare To Zero  relop=<=  OutDataTypeStr=boolean
  Gain : Gain  Gain=2
  Subtract : Sum  Inputs=+-
  dyMinStationary : Constant  Value=PcomfortStrLatDistMinSO
  latAvoidRminSO : Constant  Value=PcomfortStrRminSO
  Flg1 : Outport
  Flg2 : Outport  Port=2
  tempData1 : Outport  Port=3
  tempData2 : Outport  Port=4
  wires:
  dyRelTargetObjCalc/1 -> Add/2
  dyMinStationary/1 -> Add/1
  Add/1 -> Subtract/2, Compare To Zero1/1, tempData2/1
  Subtract/1 -> Compare To Zero/1, tempData1/1
  Compare To Zero/1 -> Flg1/1
  latAvoidRminSO/1 -> Gain/1
  Compare To Zero1/1 -> Flg2/1
  Gain/1 -> Subtract/1

== system TosAeb/FcwAebJa_Step_sys/ALG_AEBSystem/ALG_AEBController/AEB_LongTgtInfoCalc/LongObjCalcInfoCalc/AEBS_LongCollisionAssesApp/LatCollisionAvoidCalc/AccelLatAvoidCalcMO/MovingObjLatCollisionAvoidCalc/Condition1 ==
  Action Port : ActionPort  ActionPortLabel=if((u1<=0)|(u2>0))
  Bus Creator : BusCreator  Inputs=2
  Constant : Constant  Value=CNPEBSAccelNull
  Constant1 : Constant  Value=CNPEBSAccelNull
  accelLatAvoid : Outport
  wires:
  Constant/1 -> Bus Creator/1  "accelLatAvoidLeft"
  Bus Creator/1 -> accelLatAvoid/1
  Constant1/1 -> Bus Creator/2  "accelLatAvoidRight"

== system TosAeb/FcwAebJa_Step_sys/ALG_AEBSystem/ALG_AEBController/AEB_LongTgtInfoCalc/LongObjCalcInfoCalc/AEBS_LongCollisionAssesApp/LatCollisionAvoidCalc/AccelLatAvoidCalcMO/MovingObjLatCollisionAvoidCalc/Condition2 ==
  note: if TTC < 0.1
  TTC : Inport
  dy : Inport  Port=2
  vy : Inport  Port=3
  Action Port : ActionPort  ActionPortLabel=else
  If : If  IfExpression=u1
  Merge : Merge
  TTCIsZero : Subsystem
  latAvoidAccelMO : Subsystem  TreatAsAtomicUnit=on
  latAvoidAccelMoMax : Subsystem  TreatAsAtomicUnit=on
  accelLatAvoid : Outport
  wires:
  TTCIsZero/1(TTCIsZeroFlg) -> If/1
  dy/1 -> latAvoidAccelMO/1(dy)
  vy/1 -> latAvoidAccelMO/2(vy)
  Merge/1 -> accelLatAvoid/1
  latAvoidAccelMoMax/1(accelLatAvoid) -> Merge/1
  latAvoidAccelMO/1(accelLatAvoid) -> Merge/2
  If/2 -> latAvoidAccelMO/Action
  If/1 -> latAvoidAccelMoMax/Action
  TTC/1 -> latAvoidAccelMO/3(TTC), TTCIsZero/1(TTC)

== system TosAeb/FcwAebJa_Step_sys/ALG_AEBSystem/ALG_AEBController/AEB_LongTgtInfoCalc/LongObjCalcInfoCalc/AEBS_LongCollisionAssesApp/LatCollisionAvoidCalc/LatAvoidFlg4WarningJudge/accelLatAvoidThesMO/AccelLatAvoidThesCalcHmiEarly ==
  subjVel : Inport
  Action Port : ActionPort  ActionPortLabel=case [ HMISettingEarly ]:
  accelLatAvoidThesLookupTable : Lookup_n-D  NumberOfTableDimensions=1  BreakpointsForDimension1=P_AEB_LongCA_LatAvoidAccelThesWarnHmiEarlyMOX  BeginIndexSearchUsingPreviousIndexResult=on  Table=P_AEB_LongCA_LatAvoidAccelThesWarnHmiEarlyMOY  ExtrapMethod=Clip  InternalRulePriority=Speed  RndMeth=Simplest  InputSameDT=on
  accelLatAvoidThes : Outport
  wires:
  subjVel/1 -> accelLatAvoidThesLookupTable/1
  accelLatAvoidThesLookupTable/1 -> accelLatAvoidThes/1
